simulink model "isi_correlator_tvg"  (Simulink 7.3) (continued)
== system isi_correlator_tvg/fft_wideband_real/fft_direct == (continued)
      mask init: same as isi_correlator_tvg/fft_wideband_real/fft_direct/butterfly1_0
      settings: AttributesFormatString=FFTSize=7, coeff_bit_width=18
      mask StyleString: checkbox,edit,edit,edit,edit,edit,checkbox,edit,edit,edit,popup(Truncate|Round  (unbiased: +/- Inf)|Round  (unbiased: Even Values)),popup(Wrap|Saturate|Error),popup(Virtex2Pro|Virtex5),popup(Logic|Multipliers),checkbox,checkbox,checkbox
      mask CallbackString:
      | ||coeffs = eval(get_param(gcb,'Coeffs'));
      | visibs = get_param(gcb,'MaskVisibilities');
      | if length(coeffs) == 1,
      |     visibs{5} = 'off'; visibs{6} = 'off';
      |     visibs{7} = 'off';
      | else
      |     visibs{5} = 'on';
      |     visibs{6} = 'on'; visibs{7} = 'on';
      | end
      | set_param(gcb, 'MaskVisibilities', visibs);||||||||||||use_hdl = get_param(gcb,'use_hdl');
      | visibs = get_param(gcb, 'MaskVisibilities');
      | if( strcmp(use_hdl,'on')),
      |     set_param(gcb, 'use_embedded','off');
      |     visibs{16} = 'off';
      | else,
      |     visibs{16} = 'on';
      | end
      |     set_param(gcb, 'MaskVisibilities',visibs);||
  butterfly3_3 : Subsystem  AncestorBlock=casper_library/FFTs/butterfly_direct  mask(butterfly_direct){biplex=off, FFTSize=7, Coeffs=[1 17 9 25 5 21 13 29], coeff_bit_width=18, StepPeriod=0, bram_latency=3, coeffs_bram=off, input_bit_width=18, add_latency=1, mult_latency=3, quantization=Truncate, overflow=Wrap, arch=Virtex5, opt_target=Multipliers, use_hdl=off, use_embedded=on, dsp48_adders=on}
      mask prompts: biplex "Used in biplex FFT"; FFTSize "Size of FFT: (2^?)"; Coeffs "Coefficients: (0 to 2^(FFTSize-1)-1)"; coeff_bit_width "Coefficient Bit Width"; StepPeriod "Coefficient Step Period: (2^?)"; bram_latency "BRAM Latency"; coeffs_bram "Implement coefficients in BRAM"; input_bit_width "Input/Output Bit Width:"; add_latency "Add Latency"; mult_latency "Mult Latency"; quantization "Quantization Behavior"; overflow "Overflow Behavior"; arch "Architecture"; opt_target "Optimisation target"; use_hdl "Use behavioural HDL for multipliers"; use_embedded "Use embedded multipliers"; dsp48_adders "Use DSP48s for adders"
      mask init: same as isi_correlator_tvg/fft_wideband_real/fft_direct/butterfly1_0
      settings: AttributesFormatString=FFTSize=7, coeff_bit_width=18
      mask StyleString: checkbox,edit,edit,edit,edit,edit,checkbox,edit,edit,edit,popup(Truncate|Round  (unbiased: +/- Inf)|Round  (unbiased: Even Values)),popup(Wrap|Saturate|Error),popup(Virtex2Pro|Virtex5),popup(Logic|Multipliers),checkbox,checkbox,checkbox
      mask CallbackString:
      | ||coeffs = eval(get_param(gcb,'Coeffs'));
      | visibs = get_param(gcb,'MaskVisibilities');
      | if length(coeffs) == 1,
      |     visibs{5} = 'off'; visibs{6} = 'off';
      |     visibs{7} = 'off';
      | else
      |     visibs{5} = 'on';
      |     visibs{6} = 'on'; visibs{7} = 'on';
      | end
      | set_param(gcb, 'MaskVisibilities', visibs);||||||||||||use_hdl = get_param(gcb,'use_hdl');
      | visibs = get_param(gcb, 'MaskVisibilities');
      | if( strcmp(use_hdl,'on')),
      |     set_param(gcb, 'use_embedded','off');
      |     visibs{16} = 'off';
      | else,
      |     visibs{16} = 'on';
      | end
      |     set_param(gcb, 'MaskVisibilities',visibs);||
  butterfly3_4 : Subsystem  AncestorBlock=casper_library/FFTs/butterfly_direct  mask(butterfly_direct){biplex=off, FFTSize=7, Coeffs=[2 18 10 26 6 22 14 30], coeff_bit_width=18, StepPeriod=0, bram_latency=3, coeffs_bram=off, input_bit_width=18, add_latency=1, mult_latency=3, quantization=Truncate, overflow=Wrap, arch=Virtex5, opt_target=Multipliers, use_hdl=off, use_embedded=on, dsp48_adders=on}
      mask prompts: biplex "Used in biplex FFT"; FFTSize "Size of FFT: (2^?)"; Coeffs "Coefficients: (0 to 2^(FFTSize-1)-1)"; coeff_bit_width "Coefficient Bit Width"; StepPeriod "Coefficient Step Period: (2^?)"; bram_latency "BRAM Latency"; coeffs_bram "Implement coefficients in BRAM"; input_bit_width "Input/Output Bit Width:"; add_latency "Add Latency"; mult_latency "Mult Latency"; quantization "Quantization Behavior"; overflow "Overflow Behavior"; arch "Architecture"; opt_target "Optimisation target"; use_hdl "Use behavioural HDL for multipliers"; use_embedded "Use embedded multipliers"; dsp48_adders "Use DSP48s for adders"
      mask init: same as isi_correlator_tvg/fft_wideband_real/fft_direct/butterfly1_0
      settings: AttributesFormatString=FFTSize=7, coeff_bit_width=18
      mask StyleString: checkbox,edit,edit,edit,edit,edit,checkbox,edit,edit,edit,popup(Truncate|Round  (unbiased: +/- Inf)|Round  (unbiased: Even Values)),popup(Wrap|Saturate|Error),popup(Virtex2Pro|Virtex5),popup(Logic|Multipliers),checkbox,checkbox,checkbox
      mask CallbackString:
      | ||coeffs = eval(get_param(gcb,'Coeffs'));
      | visibs = get_param(gcb,'MaskVisibilities');
      | if length(coeffs) == 1,
      |     visibs{5} = 'off'; visibs{6} = 'off';
      |     visibs{7} = 'off';
      | else
      |     visibs{5} = 'on';
      |     visibs{6} = 'on'; visibs{7} = 'on';
      | end
      | set_param(gcb, 'MaskVisibilities', visibs);||||||||||||use_hdl = get_param(gcb,'use_hdl');
      | visibs = get_param(gcb, 'MaskVisibilities');
      | if( strcmp(use_hdl,'on')),
      |     set_param(gcb, 'use_embedded','off');
      |     visibs{16} = 'off';
      | else,
      |     visibs{16} = 'on';
      | end
      |     set_param(gcb, 'MaskVisibilities',visibs);||
  butterfly3_5 : Subsystem  AncestorBlock=casper_library/FFTs/butterfly_direct  mask(butterfly_direct){biplex=off, FFTSize=7, Coeffs=[2 18 10 26 6 22 14 30], coeff_bit_width=18, StepPeriod=0, bram_latency=3, coeffs_bram=off, input_bit_width=18, add_latency=1, mult_latency=3, quantization=Truncate, overflow=Wrap, arch=Virtex5, opt_target=Multipliers, use_hdl=off, use_embedded=on, dsp48_adders=on}
      mask prompts: biplex "Used in biplex FFT"; FFTSize "Size of FFT: (2^?)"; Coeffs "Coefficients: (0 to 2^(FFTSize-1)-1)"; coeff_bit_width "Coefficient Bit Width"; StepPeriod "Coefficient Step Period: (2^?)"; bram_latency "BRAM Latency"; coeffs_bram "Implement coefficients in BRAM"; input_bit_width "Input/Output Bit Width:"; add_latency "Add Latency"; mult_latency "Mult Latency"; quantization "Quantization Behavior"; overflow "Overflow Behavior"; arch "Architecture"; opt_target "Optimisation target"; use_hdl "Use behavioural HDL for multipliers"; use_embedded "Use embedded multipliers"; dsp48_adders "Use DSP48s for adders"
      mask init: same as isi_correlator_tvg/fft_wideband_real/fft_direct/butterfly1_0
      settings: AttributesFormatString=FFTSize=7, coeff_bit_width=18
      mask StyleString: checkbox,edit,edit,edit,edit,edit,checkbox,edit,edit,edit,popup(Truncate|Round  (unbiased: +/- Inf)|Round  (unbiased: Even Values)),popup(Wrap|Saturate|Error),popup(Virtex2Pro|Virtex5),popup(Logic|Multipliers),checkbox,checkbox,checkbox
      mask CallbackString:
      | ||coeffs = eval(get_param(gcb,'Coeffs'));
      | visibs = get_param(gcb,'MaskVisibilities');
      | if length(coeffs) == 1,
      |     visibs{5} = 'off'; visibs{6} = 'off';
      |     visibs{7} = 'off';
      | else
      |     visibs{5} = 'on';
      |     visibs{6} = 'on'; visibs{7} = 'on';
      | end
      | set_param(gcb, 'MaskVisibilities', visibs);||||||||||||use_hdl = get_param(gcb,'use_hdl');
      | visibs = get_param(gcb, 'MaskVisibilities');
      | if( strcmp(use_hdl,'on')),
      |     set_param(gcb, 'use_embedded','off');
      |     visibs{16} = 'off';
      | else,
      |     visibs{16} = 'on';
      | end
      |     set_param(gcb, 'MaskVisibilities',visibs);||
  butterfly3_6 : Subsystem  AncestorBlock=casper_library/FFTs/butterfly_direct  mask(butterfly_direct){biplex=off, FFTSize=7, Coeffs=[3 19 11 27 7 23 15 31], coeff_bit_width=18, StepPeriod=0, bram_latency=3, coeffs_bram=off, input_bit_width=18, add_latency=1, mult_latency=3, quantization=Truncate, overflow=Wrap, arch=Virtex5, opt_target=Multipliers, use_hdl=off, use_embedded=on, dsp48_adders=on}
      mask prompts: biplex "Used in biplex FFT"; FFTSize "Size of FFT: (2^?)"; Coeffs "Coefficients: (0 to 2^(FFTSize-1)-1)"; coeff_bit_width "Coefficient Bit Width"; StepPeriod "Coefficient Step Period: (2^?)"; bram_latency "BRAM Latency"; coeffs_bram "Implement coefficients in BRAM"; input_bit_width "Input/Output Bit Width:"; add_latency "Add Latency"; mult_latency "Mult Latency"; quantization "Quantization Behavior"; overflow "Overflow Behavior"; arch "Architecture"; opt_target "Optimisation target"; use_hdl "Use behavioural HDL for multipliers"; use_embedded "Use embedded multipliers"; dsp48_adders "Use DSP48s for adders"
      mask init: same as isi_correlator_tvg/fft_wideband_real/fft_direct/butterfly1_0
      settings: AttributesFormatString=FFTSize=7, coeff_bit_width=18
      mask StyleString: checkbox,edit,edit,edit,edit,edit,checkbox,edit,edit,edit,popup(Truncate|Round  (unbiased: +/- Inf)|Round  (unbiased: Even Values)),popup(Wrap|Saturate|Error),popup(Virtex2Pro|Virtex5),popup(Logic|Multipliers),checkbox,checkbox,checkbox
      mask CallbackString:
      | ||coeffs = eval(get_param(gcb,'Coeffs'));
      | visibs = get_param(gcb,'MaskVisibilities');
      | if length(coeffs) == 1,
      |     visibs{5} = 'off'; visibs{6} = 'off';
      |     visibs{7} = 'off';
      | else
      |     visibs{5} = 'on';
      |     visibs{6} = 'on'; visibs{7} = 'on';
      | end
      | set_param(gcb, 'MaskVisibilities', visibs);||||||||||||use_hdl = get_param(gcb,'use_hdl');
      | visibs = get_param(gcb, 'MaskVisibilities');
      | if( strcmp(use_hdl,'on')),
      |     set_param(gcb, 'use_embedded','off');
      |     visibs{16} = 'off';
      | else,
      |     visibs{16} = 'on';
      | end
      |     set_param(gcb, 'MaskVisibilities',visibs);||
  butterfly3_7 : Subsystem  AncestorBlock=casper_library/FFTs/butterfly_direct  mask(butterfly_direct){biplex=off, FFTSize=7, Coeffs=[3 19 11 27 7 23 15 31], coeff_bit_width=18, StepPeriod=0, bram_latency=3, coeffs_bram=off, input_bit_width=18, add_latency=1, mult_latency=3, quantization=Truncate, overflow=Wrap, arch=Virtex5, opt_target=Multipliers, use_hdl=off, use_embedded=on, dsp48_adders=on}
      mask prompts: biplex "Used in biplex FFT"; FFTSize "Size of FFT: (2^?)"; Coeffs "Coefficients: (0 to 2^(FFTSize-1)-1)"; coeff_bit_width "Coefficient Bit Width"; StepPeriod "Coefficient Step Period: (2^?)"; bram_latency "BRAM Latency"; coeffs_bram "Implement coefficients in BRAM"; input_bit_width "Input/Output Bit Width:"; add_latency "Add Latency"; mult_latency "Mult Latency"; quantization "Quantization Behavior"; overflow "Overflow Behavior"; arch "Architecture"; opt_target "Optimisation target"; use_hdl "Use behavioural HDL for multipliers"; use_embedded "Use embedded multipliers"; dsp48_adders "Use DSP48s for adders"
      mask init: same as isi_correlator_tvg/fft_wideband_real/fft_direct/butterfly1_0
      settings: AttributesFormatString=FFTSize=7, coeff_bit_width=18
      mask StyleString: checkbox,edit,edit,edit,edit,edit,checkbox,edit,edit,edit,popup(Truncate|Round  (unbiased: +/- Inf)|Round  (unbiased: Even Values)),popup(Wrap|Saturate|Error),popup(Virtex2Pro|Virtex5),popup(Logic|Multipliers),checkbox,checkbox,checkbox
      mask CallbackString:
      | ||coeffs = eval(get_param(gcb,'Coeffs'));
      | visibs = get_param(gcb,'MaskVisibilities');
      | if length(coeffs) == 1,
      |     visibs{5} = 'off'; visibs{6} = 'off';
      |     visibs{7} = 'off';
      | else
      |     visibs{5} = 'on';
      |     visibs{6} = 'on'; visibs{7} = 'on';
      | end
      | set_param(gcb, 'MaskVisibilities', visibs);||||||||||||use_hdl = get_param(gcb,'use_hdl');
      | visibs = get_param(gcb, 'MaskVisibilities');
      | if( strcmp(use_hdl,'on')),
      |     set_param(gcb, 'use_embedded','off');
      |     visibs{16} = 'off';
      | else,
      |     visibs{16} = 'on';
      | end
      |     set_param(gcb, 'MaskVisibilities',visibs);||
  butterfly4_0 : Subsystem  AncestorBlock=casper_library/FFTs/butterfly_direct  mask(butterfly_direct){biplex=off, FFTSize=7, Coeffs=[0 32 16 48 8 40 24 56], coeff_bit_width=18, StepPeriod=0, bram_latency=3, coeffs_bram=off, input_bit_width=18, add_latency=1, mult_latency=3, quantization=Truncate, overflow=Wrap, arch=Virtex5, opt_target=Multipliers, use_hdl=off, use_embedded=on, dsp48_adders=on}
      mask prompts: biplex "Used in biplex FFT"; FFTSize "Size of FFT: (2^?)"; Coeffs "Coefficients: (0 to 2^(FFTSize-1)-1)"; coeff_bit_width "Coefficient Bit Width"; StepPeriod "Coefficient Step Period: (2^?)"; bram_latency "BRAM Latency"; coeffs_bram "Implement coefficients in BRAM"; input_bit_width "Input/Output Bit Width:"; add_latency "Add Latency"; mult_latency "Mult Latency"; quantization "Quantization Behavior"; overflow "Overflow Behavior"; arch "Architecture"; opt_target "Optimisation target"; use_hdl "Use behavioural HDL for multipliers"; use_embedded "Use embedded multipliers"; dsp48_adders "Use DSP48s for adders"
      mask init: same as isi_correlator_tvg/fft_wideband_real/fft_direct/butterfly1_0
      settings: AttributesFormatString=FFTSize=7, coeff_bit_width=18
      mask StyleString: checkbox,edit,edit,edit,edit,edit,checkbox,edit,edit,edit,popup(Truncate|Round  (unbiased: +/- Inf)|Round  (unbiased: Even Values)),popup(Wrap|Saturate|Error),popup(Virtex2Pro|Virtex5),popup(Logic|Multipliers),checkbox,checkbox,checkbox
      mask CallbackString:
      | ||coeffs = eval(get_param(gcb,'Coeffs'));
      | visibs = get_param(gcb,'MaskVisibilities');
      | if length(coeffs) == 1,
      |     visibs{5} = 'off'; visibs{6} = 'off';
      |     visibs{7} = 'off';
      | else
      |     visibs{5} = 'on';
      |     visibs{6} = 'on'; visibs{7} = 'on';
      | end
      | set_param(gcb, 'MaskVisibilities', visibs);||||||||||||use_hdl = get_param(gcb,'use_hdl');
      | visibs = get_param(gcb, 'MaskVisibilities');
      | if( strcmp(use_hdl,'on')),
      |     set_param(gcb, 'use_embedded','off');
      |     visibs{16} = 'off';
      | else,
      |     visibs{16} = 'on';
      | end
      |     set_param(gcb, 'MaskVisibilities',visibs);||
  butterfly4_1 : Subsystem  AncestorBlock=casper_library/FFTs/butterfly_direct  mask(butterfly_direct){biplex=off, FFTSize=7, Coeffs=[1 33 17 49 9 41 25 57], coeff_bit_width=18, StepPeriod=0, bram_latency=3, coeffs_bram=off, input_bit_width=18, add_latency=1, mult_latency=3, quantization=Truncate, overflow=Wrap, arch=Virtex5, opt_target=Multipliers, use_hdl=off, use_embedded=on, dsp48_adders=on}
      mask prompts: biplex "Used in biplex FFT"; FFTSize "Size of FFT: (2^?)"; Coeffs "Coefficients: (0 to 2^(FFTSize-1)-1)"; coeff_bit_width "Coefficient Bit Width"; StepPeriod "Coefficient Step Period: (2^?)"; bram_latency "BRAM Latency"; coeffs_bram "Implement coefficients in BRAM"; input_bit_width "Input/Output Bit Width:"; add_latency "Add Latency"; mult_latency "Mult Latency"; quantization "Quantization Behavior"; overflow "Overflow Behavior"; arch "Architecture"; opt_target "Optimisation target"; use_hdl "Use behavioural HDL for multipliers"; use_embedded "Use embedded multipliers"; dsp48_adders "Use DSP48s for adders"
      mask init: same as isi_correlator_tvg/fft_wideband_real/fft_direct/butterfly1_0
      settings: AttributesFormatString=FFTSize=7, coeff_bit_width=18
      mask StyleString: checkbox,edit,edit,edit,edit,edit,checkbox,edit,edit,edit,popup(Truncate|Round  (unbiased: +/- Inf)|Round  (unbiased: Even Values)),popup(Wrap|Saturate|Error),popup(Virtex2Pro|Virtex5),popup(Logic|Multipliers),checkbox,checkbox,checkbox
      mask CallbackString:
      | ||coeffs = eval(get_param(gcb,'Coeffs'));
      | visibs = get_param(gcb,'MaskVisibilities');
      | if length(coeffs) == 1,
      |     visibs{5} = 'off'; visibs{6} = 'off';
      |     visibs{7} = 'off';
      | else
      |     visibs{5} = 'on';
      |     visibs{6} = 'on'; visibs{7} = 'on';
      | end
      | set_param(gcb, 'MaskVisibilities', visibs);||||||||||||use_hdl = get_param(gcb,'use_hdl');
      | visibs = get_param(gcb, 'MaskVisibilities');
      | if( strcmp(use_hdl,'on')),
      |     set_param(gcb, 'use_embedded','off');
      |     visibs{16} = 'off';
      | else,
      |     visibs{16} = 'on';
      | end
      |     set_param(gcb, 'MaskVisibilities',visibs);||
  butterfly4_2 : Subsystem  AncestorBlock=casper_library/FFTs/butterfly_direct  mask(butterfly_direct){biplex=off, FFTSize=7, Coeffs=[2 34 18 50 10 42 26 58], coeff_bit_width=18, StepPeriod=0, bram_latency=3, coeffs_bram=off, input_bit_width=18, add_latency=1, mult_latency=3, quantization=Truncate, overflow=Wrap, arch=Virtex5, opt_target=Multipliers, use_hdl=off, use_embedded=on, dsp48_adders=on}
      mask prompts: biplex "Used in biplex FFT"; FFTSize "Size of FFT: (2^?)"; Coeffs "Coefficients: (0 to 2^(FFTSize-1)-1)"; coeff_bit_width "Coefficient Bit Width"; StepPeriod "Coefficient Step Period: (2^?)"; bram_latency "BRAM Latency"; coeffs_bram "Implement coefficients in BRAM"; input_bit_width "Input/Output Bit Width:"; add_latency "Add Latency"; mult_latency "Mult Latency"; quantization "Quantization Behavior"; overflow "Overflow Behavior"; arch "Architecture"; opt_target "Optimisation target"; use_hdl "Use behavioural HDL for multipliers"; use_embedded "Use embedded multipliers"; dsp48_adders "Use DSP48s for adders"
      mask init: same as isi_correlator_tvg/fft_wideband_real/fft_direct/butterfly1_0
      settings: AttributesFormatString=FFTSize=7, coeff_bit_width=18
      mask StyleString: checkbox,edit,edit,edit,edit,edit,checkbox,edit,edit,edit,popup(Truncate|Round  (unbiased: +/- Inf)|Round  (unbiased: Even Values)),popup(Wrap|Saturate|Error),popup(Virtex2Pro|Virtex5),popup(Logic|Multipliers),checkbox,checkbox,checkbox
      mask CallbackString:
      | ||coeffs = eval(get_param(gcb,'Coeffs'));
      | visibs = get_param(gcb,'MaskVisibilities');
      | if length(coeffs) == 1,
      |     visibs{5} = 'off'; visibs{6} = 'off';
      |     visibs{7} = 'off';
      | else
      |     visibs{5} = 'on';
      |     visibs{6} = 'on'; visibs{7} = 'on';
      | end
      | set_param(gcb, 'MaskVisibilities', visibs);||||||||||||use_hdl = get_param(gcb,'use_hdl');
      | visibs = get_param(gcb, 'MaskVisibilities');
      | if( strcmp(use_hdl,'on')),
      |     set_param(gcb, 'use_embedded','off');
      |     visibs{16} = 'off';
      | else,
      |     visibs{16} = 'on';
      | end
      |     set_param(gcb, 'MaskVisibilities',visibs);||
  butterfly4_3 : Subsystem  AncestorBlock=casper_library/FFTs/butterfly_direct  mask(butterfly_direct){biplex=off, FFTSize=7, Coeffs=[3 35 19 51 11 43 27 59], coeff_bit_width=18, StepPeriod=0, bram_latency=3, coeffs_bram=off, input_bit_width=18, add_latency=1, mult_latency=3, quantization=Truncate, overflow=Wrap, arch=Virtex5, opt_target=Multipliers, use_hdl=off, use_embedded=on, dsp48_adders=on}
      mask prompts: biplex "Used in biplex FFT"; FFTSize "Size of FFT: (2^?)"; Coeffs "Coefficients: (0 to 2^(FFTSize-1)-1)"; coeff_bit_width "Coefficient Bit Width"; StepPeriod "Coefficient Step Period: (2^?)"; bram_latency "BRAM Latency"; coeffs_bram "Implement coefficients in BRAM"; input_bit_width "Input/Output Bit Width:"; add_latency "Add Latency"; mult_latency "Mult Latency"; quantization "Quantization Behavior"; overflow "Overflow Behavior"; arch "Architecture"; opt_target "Optimisation target"; use_hdl "Use behavioural HDL for multipliers"; use_embedded "Use embedded multipliers"; dsp48_adders "Use DSP48s for adders"
      mask init: same as isi_correlator_tvg/fft_wideband_real/fft_direct/butterfly1_0
      settings: AttributesFormatString=FFTSize=7, coeff_bit_width=18
      mask StyleString: checkbox,edit,edit,edit,edit,edit,checkbox,edit,edit,edit,popup(Truncate|Round  (unbiased: +/- Inf)|Round  (unbiased: Even Values)),popup(Wrap|Saturate|Error),popup(Virtex2Pro|Virtex5),popup(Logic|Multipliers),checkbox,checkbox,checkbox
      mask CallbackString:
      | ||coeffs = eval(get_param(gcb,'Coeffs'));
      | visibs = get_param(gcb,'MaskVisibilities');
      | if length(coeffs) == 1,
      |     visibs{5} = 'off'; visibs{6} = 'off';
      |     visibs{7} = 'off';
      | else
      |     visibs{5} = 'on';
      |     visibs{6} = 'on'; visibs{7} = 'on';
      | end
      | set_param(gcb, 'MaskVisibilities', visibs);||||||||||||use_hdl = get_param(gcb,'use_hdl');
      | visibs = get_param(gcb, 'MaskVisibilities');
      | if( strcmp(use_hdl,'on')),
      |     set_param(gcb, 'use_embedded','off');
      |     visibs{16} = 'off';
      | else,
      |     visibs{16} = 'on';
      | end
      |     set_param(gcb, 'MaskVisibilities',visibs);||
  butterfly4_4 : Subsystem  AncestorBlock=casper_library/FFTs/butterfly_direct  mask(butterfly_direct){biplex=off, FFTSize=7, Coeffs=[4 36 20 52 12 44 28 60], coeff_bit_width=18, StepPeriod=0, bram_latency=3, coeffs_bram=off, input_bit_width=18, add_latency=1, mult_latency=3, quantization=Truncate, overflow=Wrap, arch=Virtex5, opt_target=Multipliers, use_hdl=off, use_embedded=on, dsp48_adders=on}
      mask prompts: biplex "Used in biplex FFT"; FFTSize "Size of FFT: (2^?)"; Coeffs "Coefficients: (0 to 2^(FFTSize-1)-1)"; coeff_bit_width "Coefficient Bit Width"; StepPeriod "Coefficient Step Period: (2^?)"; bram_latency "BRAM Latency"; coeffs_bram "Implement coefficients in BRAM"; input_bit_width "Input/Output Bit Width:"; add_latency "Add Latency"; mult_latency "Mult Latency"; quantization "Quantization Behavior"; overflow "Overflow Behavior"; arch "Architecture"; opt_target "Optimisation target"; use_hdl "Use behavioural HDL for multipliers"; use_embedded "Use embedded multipliers"; dsp48_adders "Use DSP48s for adders"
      mask init: same as isi_correlator_tvg/fft_wideband_real/fft_direct/butterfly1_0
      settings: AttributesFormatString=FFTSize=7, coeff_bit_width=18
      mask StyleString: checkbox,edit,edit,edit,edit,edit,checkbox,edit,edit,edit,popup(Truncate|Round  (unbiased: +/- Inf)|Round  (unbiased: Even Values)),popup(Wrap|Saturate|Error),popup(Virtex2Pro|Virtex5),popup(Logic|Multipliers),checkbox,checkbox,checkbox
      mask CallbackString:
      | ||coeffs = eval(get_param(gcb,'Coeffs'));
      | visibs = get_param(gcb,'MaskVisibilities');
      | if length(coeffs) == 1,
      |     visibs{5} = 'off'; visibs{6} = 'off';
      |     visibs{7} = 'off';
      | else
      |     visibs{5} = 'on';
      |     visibs{6} = 'on'; visibs{7} = 'on';
      | end
      | set_param(gcb, 'MaskVisibilities', visibs);||||||||||||use_hdl = get_param(gcb,'use_hdl');
      | visibs = get_param(gcb, 'MaskVisibilities');
      | if( strcmp(use_hdl,'on')),
      |     set_param(gcb, 'use_embedded','off');
      |     visibs{16} = 'off';
      | else,
      |     visibs{16} = 'on';
      | end
      |     set_param(gcb, 'MaskVisibilities',visibs);||
  butterfly4_5 : Subsystem  AncestorBlock=casper_library/FFTs/butterfly_direct  mask(butterfly_direct){biplex=off, FFTSize=7, Coeffs=[5 37 21 53 13 45 29 61], coeff_bit_width=18, StepPeriod=0, bram_latency=3, coeffs_bram=off, input_bit_width=18, add_latency=1, mult_latency=3, quantization=Truncate, overflow=Wrap, arch=Virtex5, opt_target=Multipliers, use_hdl=off, use_embedded=on, dsp48_adders=on}
      mask prompts: biplex "Used in biplex FFT"; FFTSize "Size of FFT: (2^?)"; Coeffs "Coefficients: (0 to 2^(FFTSize-1)-1)"; coeff_bit_width "Coefficient Bit Width"; StepPeriod "Coefficient Step Period: (2^?)"; bram_latency "BRAM Latency"; coeffs_bram "Implement coefficients in BRAM"; input_bit_width "Input/Output Bit Width:"; add_latency "Add Latency"; mult_latency "Mult Latency"; quantization "Quantization Behavior"; overflow "Overflow Behavior"; arch "Architecture"; opt_target "Optimisation target"; use_hdl "Use behavioural HDL for multipliers"; use_embedded "Use embedded multipliers"; dsp48_adders "Use DSP48s for adders"
      mask init: same as isi_correlator_tvg/fft_wideband_real/fft_direct/butterfly1_0
      settings: AttributesFormatString=FFTSize=7, coeff_bit_width=18
      mask StyleString: checkbox,edit,edit,edit,edit,edit,checkbox,edit,edit,edit,popup(Truncate|Round  (unbiased: +/- Inf)|Round  (unbiased: Even Values)),popup(Wrap|Saturate|Error),popup(Virtex2Pro|Virtex5),popup(Logic|Multipliers),checkbox,checkbox,checkbox
      mask CallbackString:
      | ||coeffs = eval(get_param(gcb,'Coeffs'));
      | visibs = get_param(gcb,'MaskVisibilities');
      | if length(coeffs) == 1,
      |     visibs{5} = 'off'; visibs{6} = 'off';
      |     visibs{7} = 'off';
      | else
      |     visibs{5} = 'on';
      |     visibs{6} = 'on'; visibs{7} = 'on';
      | end
      | set_param(gcb, 'MaskVisibilities', visibs);||||||||||||use_hdl = get_param(gcb,'use_hdl');
      | visibs = get_param(gcb, 'MaskVisibilities');
      | if( strcmp(use_hdl,'on')),
      |     set_param(gcb, 'use_embedded','off');
      |     visibs{16} = 'off';
      | else,
      |     visibs{16} = 'on';
      | end
      |     set_param(gcb, 'MaskVisibilities',visibs);||
  butterfly4_6 : Subsystem  AncestorBlock=casper_library/FFTs/butterfly_direct  mask(butterfly_direct){biplex=off, FFTSize=7, Coeffs=[6 38 22 54 14 46 30 62], coeff_bit_width=18, StepPeriod=0, bram_latency=3, coeffs_bram=off, input_bit_width=18, add_latency=1, mult_latency=3, quantization=Truncate, overflow=Wrap, arch=Virtex5, opt_target=Multipliers, use_hdl=off, use_embedded=on, dsp48_adders=on}
      mask prompts: biplex "Used in biplex FFT"; FFTSize "Size of FFT: (2^?)"; Coeffs "Coefficients: (0 to 2^(FFTSize-1)-1)"; coeff_bit_width "Coefficient Bit Width"; StepPeriod "Coefficient Step Period: (2^?)"; bram_latency "BRAM Latency"; coeffs_bram "Implement coefficients in BRAM"; input_bit_width "Input/Output Bit Width:"; add_latency "Add Latency"; mult_latency "Mult Latency"; quantization "Quantization Behavior"; overflow "Overflow Behavior"; arch "Architecture"; opt_target "Optimisation target"; use_hdl "Use behavioural HDL for multipliers"; use_embedded "Use embedded multipliers"; dsp48_adders "Use DSP48s for adders"
      mask init: same as isi_correlator_tvg/fft_wideband_real/fft_direct/butterfly1_0
      settings: AttributesFormatString=FFTSize=7, coeff_bit_width=18
      mask StyleString: checkbox,edit,edit,edit,edit,edit,checkbox,edit,edit,edit,popup(Truncate|Round  (unbiased: +/- Inf)|Round  (unbiased: Even Values)),popup(Wrap|Saturate|Error),popup(Virtex2Pro|Virtex5),popup(Logic|Multipliers),checkbox,checkbox,checkbox
      mask CallbackString:
      | ||coeffs = eval(get_param(gcb,'Coeffs'));
      | visibs = get_param(gcb,'MaskVisibilities');
      | if length(coeffs) == 1,
      |     visibs{5} = 'off'; visibs{6} = 'off';
      |     visibs{7} = 'off';
      | else
      |     visibs{5} = 'on';
      |     visibs{6} = 'on'; visibs{7} = 'on';
      | end
      | set_param(gcb, 'MaskVisibilities', visibs);||||||||||||use_hdl = get_param(gcb,'use_hdl');
      | visibs = get_param(gcb, 'MaskVisibilities');
      | if( strcmp(use_hdl,'on')),
      |     set_param(gcb, 'use_embedded','off');
      |     visibs{16} = 'off';
      | else,
      |     visibs{16} = 'on';
      | end
      |     set_param(gcb, 'MaskVisibilities',visibs);||
  butterfly4_7 : Subsystem  AncestorBlock=casper_library/FFTs/butterfly_direct  mask(butterfly_direct){biplex=off, FFTSize=7, Coeffs=[7 39 23 55 15 47 31 63], coeff_bit_width=18, StepPeriod=0, bram_latency=3, coeffs_bram=off, input_bit_width=18, add_latency=1, mult_latency=3, quantization=Truncate, overflow=Wrap, arch=Virtex5, opt_target=Multipliers, use_hdl=off, use_embedded=on, dsp48_adders=on}
      mask prompts: biplex "Used in biplex FFT"; FFTSize "Size of FFT: (2^?)"; Coeffs "Coefficients: (0 to 2^(FFTSize-1)-1)"; coeff_bit_width "Coefficient Bit Width"; StepPeriod "Coefficient Step Period: (2^?)"; bram_latency "BRAM Latency"; coeffs_bram "Implement coefficients in BRAM"; input_bit_width "Input/Output Bit Width:"; add_latency "Add Latency"; mult_latency "Mult Latency"; quantization "Quantization Behavior"; overflow "Overflow Behavior"; arch "Architecture"; opt_target "Optimisation target"; use_hdl "Use behavioural HDL for multipliers"; use_embedded "Use embedded multipliers"; dsp48_adders "Use DSP48s for adders"
      mask init: same as isi_correlator_tvg/fft_wideband_real/fft_direct/butterfly1_0
      settings: AttributesFormatString=FFTSize=7, coeff_bit_width=18
      mask StyleString: checkbox,edit,edit,edit,edit,edit,checkbox,edit,edit,edit,popup(Truncate|Round  (unbiased: +/- Inf)|Round  (unbiased: Even Values)),popup(Wrap|Saturate|Error),popup(Virtex2Pro|Virtex5),popup(Logic|Multipliers),checkbox,checkbox,checkbox
      mask CallbackString:
      | ||coeffs = eval(get_param(gcb,'Coeffs'));
      | visibs = get_param(gcb,'MaskVisibilities');
      | if length(coeffs) == 1,
      |     visibs{5} = 'off'; visibs{6} = 'off';
      |     visibs{7} = 'off';
      | else
      |     visibs{5} = 'on';
      |     visibs{6} = 'on'; visibs{7} = 'on';
      | end
      | set_param(gcb, 'MaskVisibilities', visibs);||||||||||||use_hdl = get_param(gcb,'use_hdl');
      | visibs = get_param(gcb, 'MaskVisibilities');
      | if( strcmp(use_hdl,'on')),
      |     set_param(gcb, 'use_embedded','off');
      |     visibs{16} = 'off';
      | else,
      |     visibs{16} = 'on';
      | end
      |     set_param(gcb, 'MaskVisibilities',visibs);||
  node0_{0..15} (16 alike) : xbsIndex_r4/Delay  en=off  latency=0  dbl_ovrd=off  reg_retiming=off  block_type=delay
      node0_0 settings: xl_area=[0, 0, 0, 0, 0, 0, 0]  block_version=9.1.01
      node0_1 settings: xl_area=[0, 0, 0, 0, 0, 0, 0]  block_version=9.1.01
      node0_10 settings: block_version=11.3
      node0_11 settings: block_version=11.3
      node0_12 settings: block_version=11.3
      node0_13 settings: block_version=11.3
      node0_14 settings: block_version=11.3
      node0_15 settings: block_version=11.3
      node0_4 settings: block_version=11.3
      node0_5 settings: block_version=11.3
      node0_6 settings: block_version=11.3
      node0_7 settings: block_version=11.3
      node0_8 settings: block_version=11.3
      node0_9 settings: block_version=11.3
  node1_{0..15} (16 alike) : xbsIndex_r4/Delay  en=off  latency=0  dbl_ovrd=off  reg_retiming=off  block_type=delay
      node1_0 settings: xl_area=[0, 0, 0, 0, 0, 0, 0]  block_version=9.1.01
      node1_1 settings: xl_area=[0, 0, 0, 0, 0, 0, 0]  block_version=9.1.01
      node1_10 settings: block_version=11.3
      node1_11 settings: block_version=11.3
      node1_12 settings: block_version=11.3
      node1_13 settings: block_version=11.3
      node1_14 settings: block_version=11.3
      node1_15 settings: block_version=11.3
      node1_4 settings: block_version=11.3
      node1_5 settings: block_version=11.3
      node1_6 settings: block_version=11.3
      node1_7 settings: block_version=11.3
      node1_8 settings: block_version=11.3
      node1_9 settings: block_version=11.3
  node2_{0..15} (16 alike) : xbsIndex_r4/Delay  en=off  latency=0  dbl_ovrd=off  reg_retiming=off  block_type=delay
      node2_10 settings: block_version=11.3
      node2_11 settings: block_version=11.3
      node2_12 settings: block_version=11.3
      node2_13 settings: block_version=11.3
      node2_14 settings: block_version=11.3
      node2_15 settings: block_version=11.3
      node2_4 settings: block_version=11.3
      node2_5 settings: block_version=11.3
      node2_6 settings: block_version=11.3
      node2_7 settings: block_version=11.3
      node2_8 settings: block_version=11.3
      node2_9 settings: block_version=11.3
  node3_{0..15} (16 alike) : xbsIndex_r4/Delay  en=off  latency=0  dbl_ovrd=off  reg_retiming=off  block_type=delay
      settings: block_version=11.3
  node4_{0..15} (16 alike) : xbsIndex_r4/Delay  en=off  latency=0  dbl_ovrd=off  reg_retiming=off  block_type=delay
      settings: block_version=11.3
  of_{1..4} (4 alike) : xbsIndex_r4/Logical  logical_function=OR  inputs=8  en=off  latency=1  precision=Full  arith_type=Unsigned  n_bits=16  bin_pt=0  align_bp=on  dbl_ovrd=off  block_type=logical
      of_3 settings: block_version=11.3
      of_4 settings: block_version=11.3
  of_or : xbsIndex_r4/Logical  logical_function=OR  inputs=4  en=off  latency=0  precision=Full  arith_type=Unsigned  n_bits=16  bin_pt=0  align_bp=on  dbl_ovrd=off  block_type=logical
  slice0 : xbsIndex_r4/Slice  nbits=1  boolean_output=on  mode=Lower Bit Location + Width  bit1=0  base1=MSB of Input  bit0=0  base0=LSB of Input  dbl_ovrd=off  block_type=slice
      settings: infoedit="Extracts a given range of bits from each input sample and presents it at the output.  The output type is ordinarily unsigned with binary point at zero, but can be Boolean when the slice is one bit wide.<P><P>Hardware notes: In hardware this block costs nothing."  block_version=9.1.01
  slice1 : xbsIndex_r4/Slice  nbits=1  boolean_output=on  mode=Lower Bit Location + Width  bit1=0  base1=MSB of Input  bit0=1  base0=LSB of Input  dbl_ovrd=off  block_type=slice
      settings: infoedit="Extracts a given range of bits from each input sample and presents it at the output.  The output type is ordinarily unsigned with binary point at zero, but can be Boolean when the slice is one bit wide.<P><P>Hardware notes: In hardware this block costs nothing."  block_version=10.1.3
  slice2 : xbsIndex_r4/Slice  nbits=1  boolean_output=on  mode=Lower Bit Location + Width  bit1=0  base1=MSB of Input  bit0=2  base0=LSB of Input  dbl_ovrd=off  block_type=slice
      settings: block_version=11.3
  slice3 : xbsIndex_r4/Slice  nbits=1  boolean_output=on  mode=Lower Bit Location + Width  bit1=0  base1=MSB of Input  bit0=3  base0=LSB of Input  dbl_ovrd=off  block_type=slice
      settings: block_version=11.3
  sync_out : Outport  OutDataType=sfix(16)  OutScaling=2^0
  out0 : Outport  Port=2  OutDataType=sfix(16)  OutScaling=2^0
  out1 : Outport  Port=3  OutDataType=sfix(16)  OutScaling=2^0
  out2 : Outport  Port=4
  out3 : Outport  Port=5
  out4 : Outport  Port=6
  out5 : Outport  Port=7
  out6 : Outport  Port=8
  out7 : Outport  Port=9
  out8 : Outport  Port=10
  out9 : Outport  Port=11
  out10 : Outport  Port=12
  out11 : Outport  Port=13
  out12 : Outport  Port=14
  out13 : Outport  Port=15
  out14 : Outport  Port=16
  out15 : Outport  Port=17
  of : Outport  Port=18
  wires:
  of_or/1 -> of/1
  in0/1 -> node0_0/1
  in1/1 -> node0_1/1
  in2/1 -> node0_2/1
  in3/1 -> node0_3/1
  in4/1 -> node0_4/1
  in5/1 -> node0_5/1
  in6/1 -> node0_6/1
  in7/1 -> node0_7/1
  in8/1 -> node0_8/1
  in9/1 -> node0_9/1
  in10/1 -> node0_10/1
  in11/1 -> node0_11/1
  in12/1 -> node0_12/1
  in13/1 -> node0_13/1
  in14/1 -> node0_14/1
  in15/1 -> node0_15/1
  shift/1 -> slice0/1, slice1/1, slice2/1, slice3/1
  node4_0/1 -> out0/1
  node4_1/1 -> out8/1
  node4_2/1 -> out4/1
  node4_3/1 -> out12/1
  node4_4/1 -> out2/1
  node4_5/1 -> out10/1
  node4_6/1 -> out6/1
  node4_7/1 -> out14/1
  node4_8/1 -> out1/1
  node4_9/1 -> out9/1
  node4_10/1 -> out5/1
  node4_11/1 -> out13/1
  node4_12/1 -> out3/1
  node4_13/1 -> out11/1
  node4_14/1 -> out7/1
  node4_15/1 -> out15/1
  of_1/1 -> of_or/1
  node0_0/1 -> butterfly1_0/1(a)
  node0_8/1 -> butterfly1_0/2(b)
  butterfly1_0/1(a+bw) -> node1_0/1
  butterfly1_0/2(a-bw) -> node1_8/1
  sync/1 -> butterfly1_0/3(sync), butterfly1_1/3(sync), butterfly1_2/3(sync), butterfly1_3/3(sync), butterfly1_4/3(sync), butterfly1_5/3(sync), butterfly1_6/3(sync), butterfly1_7/3(sync)
  slice0/1 -> butterfly1_0/4(shift), butterfly1_1/4(shift), butterfly1_2/4(shift), butterfly1_3/4(shift), butterfly1_4/4(shift), butterfly1_5/4(shift), butterfly1_6/4(shift), butterfly1_7/4(shift)
  butterfly1_0/3(of) -> of_1/1
  node0_1/1 -> butterfly1_1/1(a)
  node0_9/1 -> butterfly1_1/2(b)
  butterfly1_1/1(a+bw) -> node1_1/1
  butterfly1_1/2(a-bw) -> node1_9/1
  butterfly1_1/3(of) -> of_1/2
  node0_2/1 -> butterfly1_2/1(a)
  node0_10/1 -> butterfly1_2/2(b)
  butterfly1_2/1(a+bw) -> node1_2/1
  butterfly1_2/2(a-bw) -> node1_10/1
  butterfly1_2/3(of) -> of_1/3
  node0_3/1 -> butterfly1_3/1(a)
  node0_11/1 -> butterfly1_3/2(b)
  butterfly1_3/1(a+bw) -> node1_3/1
  butterfly1_3/2(a-bw) -> node1_11/1
  butterfly1_3/3(of) -> of_1/4
  node0_4/1 -> butterfly1_4/1(a)
  node0_12/1 -> butterfly1_4/2(b)
  butterfly1_4/1(a+bw) -> node1_4/1
  butterfly1_4/2(a-bw) -> node1_12/1
  butterfly1_4/3(of) -> of_1/5
  node0_5/1 -> butterfly1_5/1(a)
  node0_13/1 -> butterfly1_5/2(b)
  butterfly1_5/1(a+bw) -> node1_5/1
  butterfly1_5/2(a-bw) -> node1_13/1
  butterfly1_5/3(of) -> of_1/6
  node0_6/1 -> butterfly1_6/1(a)
  node0_14/1 -> butterfly1_6/2(b)
  butterfly1_6/1(a+bw) -> node1_6/1
  butterfly1_6/2(a-bw) -> node1_14/1
  butterfly1_6/3(of) -> of_1/7
  node0_7/1 -> butterfly1_7/1(a)
  node0_15/1 -> butterfly1_7/2(b)
  butterfly1_7/1(a+bw) -> node1_7/1
  butterfly1_7/2(a-bw) -> node1_15/1
  butterfly1_7/3(of) -> of_1/8
  of_2/1 -> of_or/2
  node1_0/1 -> butterfly2_0/1(a)
  node1_4/1 -> butterfly2_0/2(b)
  butterfly2_0/1(a+bw) -> node2_0/1
  butterfly2_0/2(a-bw) -> node2_4/1
  butterfly1_0/4(sync_out) -> butterfly2_0/3(sync)
  slice1/1 -> butterfly2_0/4(shift), butterfly2_1/4(shift), butterfly2_2/4(shift), butterfly2_3/4(shift), butterfly2_4/4(shift), butterfly2_5/4(shift), butterfly2_6/4(shift), butterfly2_7/4(shift)
  butterfly2_0/3(of) -> of_2/1
  node1_1/1 -> butterfly2_1/1(a)
  node1_5/1 -> butterfly2_1/2(b)
  butterfly2_1/1(a+bw) -> node2_1/1
  butterfly2_1/2(a-bw) -> node2_5/1
  butterfly1_1/4(sync_out) -> butterfly2_1/3(sync)
  butterfly2_1/3(of) -> of_2/2
  node1_2/1 -> butterfly2_2/1(a)
  node1_6/1 -> butterfly2_2/2(b)
  butterfly2_2/1(a+bw) -> node2_2/1
  butterfly2_2/2(a-bw) -> node2_6/1
  butterfly1_2/4(sync_out) -> butterfly2_2/3(sync)
  butterfly2_2/3(of) -> of_2/3
  node1_3/1 -> butterfly2_3/1(a)
  node1_7/1 -> butterfly2_3/2(b)
  butterfly2_3/1(a+bw) -> node2_3/1
  butterfly2_3/2(a-bw) -> node2_7/1
  butterfly1_3/4(sync_out) -> butterfly2_3/3(sync)
  butterfly2_3/3(of) -> of_2/4
  node1_8/1 -> butterfly2_4/1(a)
  node1_12/1 -> butterfly2_4/2(b)
  butterfly2_4/1(a+bw) -> node2_8/1
  butterfly2_4/2(a-bw) -> node2_12/1
  butterfly1_4/4(sync_out) -> butterfly2_4/3(sync)
  butterfly2_4/3(of) -> of_2/5
  node1_9/1 -> butterfly2_5/1(a)
  node1_13/1 -> butterfly2_5/2(b)
  butterfly2_5/1(a+bw) -> node2_9/1
  butterfly2_5/2(a-bw) -> node2_13/1
  butterfly1_5/4(sync_out) -> butterfly2_5/3(sync)
  butterfly2_5/3(of) -> of_2/6
  node1_10/1 -> butterfly2_6/1(a)
  node1_14/1 -> butterfly2_6/2(b)
  butterfly2_6/1(a+bw) -> node2_10/1
  butterfly2_6/2(a-bw) -> node2_14/1
  butterfly1_6/4(sync_out) -> butterfly2_6/3(sync)
  butterfly2_6/3(of) -> of_2/7
  node1_11/1 -> butterfly2_7/1(a)
  node1_15/1 -> butterfly2_7/2(b)
  butterfly2_7/1(a+bw) -> node2_11/1
  butterfly2_7/2(a-bw) -> node2_15/1
  butterfly1_7/4(sync_out) -> butterfly2_7/3(sync)
  butterfly2_7/3(of) -> of_2/8
  of_3/1 -> of_or/3
  node2_0/1 -> butterfly3_0/1(a)
  node2_2/1 -> butterfly3_0/2(b)
  butterfly3_0/1(a+bw) -> node3_0/1
  butterfly3_0/2(a-bw) -> node3_2/1
  butterfly2_0/4(sync_out) -> butterfly3_0/3(sync)
  slice2/1 -> butterfly3_0/4(shift), butterfly3_1/4(shift), butterfly3_2/4(shift), butterfly3_3/4(shift), butterfly3_4/4(shift), butterfly3_5/4(shift), butterfly3_6/4(shift), butterfly3_7/4(shift)
  butterfly3_0/3(of) -> of_3/1
  node2_1/1 -> butterfly3_1/1(a)
  node2_3/1 -> butterfly3_1/2(b)
  butterfly3_1/1(a+bw) -> node3_1/1
  butterfly3_1/2(a-bw) -> node3_3/1
  butterfly2_1/4(sync_out) -> butterfly3_1/3(sync)
  butterfly3_1/3(of) -> of_3/2
  node2_4/1 -> butterfly3_2/1(a)
  node2_6/1 -> butterfly3_2/2(b)
  butterfly3_2/1(a+bw) -> node3_4/1
  butterfly3_2/2(a-bw) -> node3_6/1
  butterfly2_2/4(sync_out) -> butterfly3_2/3(sync)
  butterfly3_2/3(of) -> of_3/3
  node2_5/1 -> butterfly3_3/1(a)
  node2_7/1 -> butterfly3_3/2(b)
  butterfly3_3/1(a+bw) -> node3_5/1
  butterfly3_3/2(a-bw) -> node3_7/1
  butterfly2_3/4(sync_out) -> butterfly3_3/3(sync)
  butterfly3_3/3(of) -> of_3/4
  node2_8/1 -> butterfly3_4/1(a)
  node2_10/1 -> butterfly3_4/2(b)
  butterfly3_4/1(a+bw) -> node3_8/1
  butterfly3_4/2(a-bw) -> node3_10/1
  butterfly2_4/4(sync_out) -> butterfly3_4/3(sync)
  butterfly3_4/3(of) -> of_3/5
  node2_9/1 -> butterfly3_5/1(a)
  node2_11/1 -> butterfly3_5/2(b)
  butterfly3_5/1(a+bw) -> node3_9/1
  butterfly3_5/2(a-bw) -> node3_11/1
  butterfly2_5/4(sync_out) -> butterfly3_5/3(sync)
  butterfly3_5/3(of) -> of_3/6
  node2_12/1 -> butterfly3_6/1(a)
  node2_14/1 -> butterfly3_6/2(b)
  butterfly3_6/1(a+bw) -> node3_12/1
  butterfly3_6/2(a-bw) -> node3_14/1
  butterfly2_6/4(sync_out) -> butterfly3_6/3(sync)
  butterfly3_6/3(of) -> of_3/7
  node2_13/1 -> butterfly3_7/1(a)
  node2_15/1 -> butterfly3_7/2(b)
  butterfly3_7/1(a+bw) -> node3_13/1
  butterfly3_7/2(a-bw) -> node3_15/1
  butterfly2_7/4(sync_out) -> butterfly3_7/3(sync)
  butterfly3_7/3(of) -> of_3/8
  of_4/1 -> of_or/4
  node3_0/1 -> butterfly4_0/1(a)
  node3_1/1 -> butterfly4_0/2(b)
  butterfly4_0/1(a+bw) -> node4_0/1
  butterfly4_0/2(a-bw) -> node4_1/1
  butterfly3_0/4(sync_out) -> butterfly4_0/3(sync)
  butterfly4_0/4(sync_out) -> sync_out/1
  slice3/1 -> butterfly4_0/4(shift), butterfly4_1/4(shift), butterfly4_2/4(shift), butterfly4_3/4(shift), butterfly4_4/4(shift), butterfly4_5/4(shift), butterfly4_6/4(shift), butterfly4_7/4(shift)
  butterfly4_0/3(of) -> of_4/1
  node3_2/1 -> butterfly4_1/1(a)
  node3_3/1 -> butterfly4_1/2(b)
  butterfly4_1/1(a+bw) -> node4_2/1
  butterfly4_1/2(a-bw) -> node4_3/1
  butterfly3_1/4(sync_out) -> butterfly4_1/3(sync)
  butterfly4_1/3(of) -> of_4/2
  node3_4/1 -> butterfly4_2/1(a)
  node3_5/1 -> butterfly4_2/2(b)
  butterfly4_2/1(a+bw) -> node4_4/1
  butterfly4_2/2(a-bw) -> node4_5/1
  butterfly3_2/4(sync_out) -> butterfly4_2/3(sync)
  butterfly4_2/3(of) -> of_4/3
  node3_6/1 -> butterfly4_3/1(a)
  node3_7/1 -> butterfly4_3/2(b)
  butterfly4_3/1(a+bw) -> node4_6/1
  butterfly4_3/2(a-bw) -> node4_7/1
  butterfly3_3/4(sync_out) -> butterfly4_3/3(sync)
  butterfly4_3/3(of) -> of_4/4
  node3_8/1 -> butterfly4_4/1(a)
  node3_9/1 -> butterfly4_4/2(b)
  butterfly4_4/1(a+bw) -> node4_8/1
  butterfly4_4/2(a-bw) -> node4_9/1
  butterfly3_4/4(sync_out) -> butterfly4_4/3(sync)
  butterfly4_4/3(of) -> of_4/5
  node3_10/1 -> butterfly4_5/1(a)
  node3_11/1 -> butterfly4_5/2(b)
  butterfly4_5/1(a+bw) -> node4_10/1
  butterfly4_5/2(a-bw) -> node4_11/1
  butterfly3_5/4(sync_out) -> butterfly4_5/3(sync)
  butterfly4_5/3(of) -> of_4/6
  node3_12/1 -> butterfly4_6/1(a)
  node3_13/1 -> butterfly4_6/2(b)
  butterfly4_6/1(a+bw) -> node4_12/1
  butterfly4_6/2(a-bw) -> node4_13/1
  butterfly3_6/4(sync_out) -> butterfly4_6/3(sync)
  butterfly4_6/3(of) -> of_4/7
  node3_14/1 -> butterfly4_7/1(a)
  node3_15/1 -> butterfly4_7/2(b)
  butterfly4_7/1(a+bw) -> node4_14/1
  butterfly4_7/2(a-bw) -> node4_15/1
  butterfly3_7/4(sync_out) -> butterfly4_7/3(sync)
  butterfly4_7/3(of) -> of_4/8
